simulink model "AirPmCtrl"  (R2022b)
settings: Solver=FixedStepDiscrete  StartTime=0.0  StopTime=10.0  FixedStep=1  RelTol=1e-3
settings Model: Creator=blue.ge  LastModifiedBy=blue.ge
settings DataIOCC: SaveFormat=StructureWithTime
settings OptimizationCC: DefaultParameterBehavior=Inlined  EnhancedBackFolding=on  PassReuseOutputArgsAs=Individual arguments  ZeroExternalMemoryAtStartup=off  ZeroInternalMemoryAtStartup=off  OptimizeBlockOrder=speed  DifferentSizesBufferReuse=on  OptimizationCustomize=off  EfficientTunableParamExpr=on
settings DebuggingCC: ParameterTunabilityLossMsg=error
settings ModelReferenceCC: ModelReferenceNumInstancesAllowed=Single
settings SFSimCC: MATLABDynamicMemAlloc=off
settings RTWCC: Description=AUTOSAR  SystemTargetFile=autosar.tlc  GenCodeOnly=on  TemplateMakefile=ert_default_tmf
settings CodeAppCC: ForceParamTrailComments=on  IgnoreCustomStorageClasses=off  OperatorAnnotations=on  SimulinkDataObjDesc=on  SFDataObjDesc=on  InsertBlockDesc=on
settings STFCustomTargetCC: TargetLangStandard=C99 (ISO)  MultiwordLength=256  ParMdlRefBuildCompliant=on  CompOptLevelCompliant=on  IncludeMdlTerminateFcn=off  CombineOutputUpdateFcns=on  SuppressErrorStatus=on  ERTCustomFileBanners=on  SupportNonFinite=off  SupportComplex=off  SupportContinuousTime=off  SupportNonInlinedSFcns=off  RemoveResetFunc=on  MATLABClassNameForMDSCustomization=Simulink.SoftwareTarget.ERTCustomization  AutosarCompliant=on  UseToolchainInfoCompliant=on  LookupTableObjectStructAxisOrder=2,1,3,4,...  LUTObjectStructOrderEvenSpacing=Size,Table,Breakpoints  SystemTargetFile=autosar.tlc
settings ERTTargetCC: TargetLangStandard=C99 (ISO)  ConcurrentExecutionCompliant=off  IncludeMdlTerminateFcn=off  SuppressErrorStatus=on  SupportNonFinite=off  SupportComplex=off  ModelStepFunctionPrototypeControlCompliant=off  CPPClassGenCompliant=off  AutosarCompliant=on  LookupTableObjectStructAxisOrder=2,1,3,4,...  LUTObjectStructOrderEvenSpacing=Size,Table,Breakpoints  InlinedParameterPlacement=NonHierarchical  ERTCustomFileTemplate=example_file_process.tlc

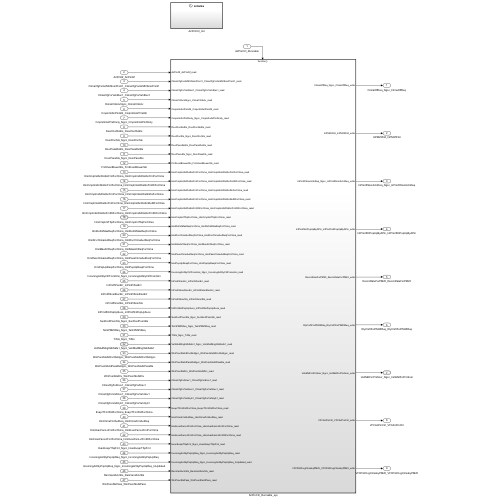
[thumbnail: Simulink's stored preview of the top-level diagram]
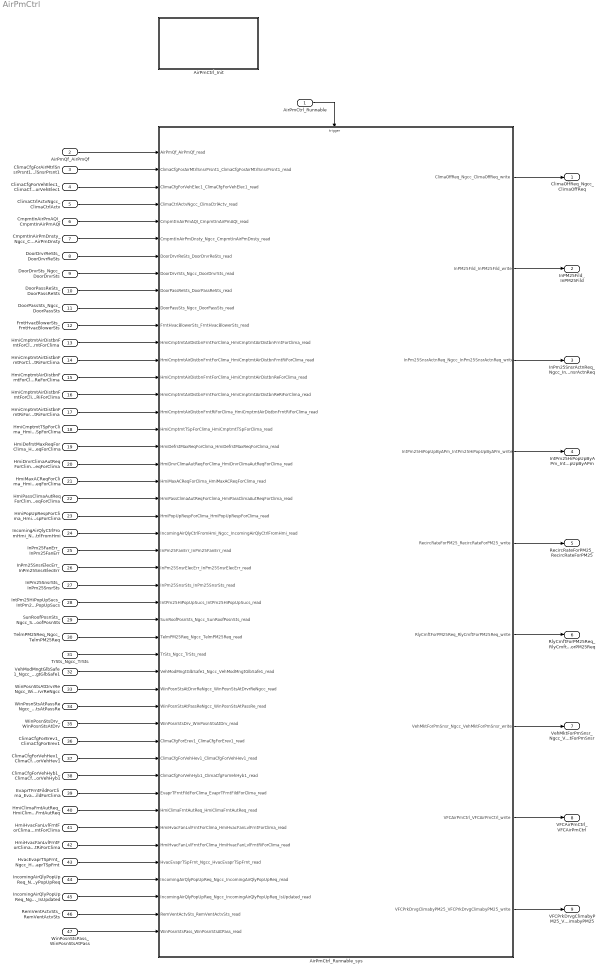
== system AirPmCtrl ==
  AirPmCtrl_Runnable : Inport  SampleTime=1  OutputFunctionCall=on
  AirPmQf_AirPmQf : Inport  Port=2  OutDataTypeStr=Enum: AirPmQf  PortDimensions=1
  ClimaCfgForAirMtrlSnsrPrsnt1_ClimaCfgForAirMtrlSnsrPrsnt1 : Inport  Port=3  OutDataTypeStr=Enum: CarCfgForAirMtrlSnsrGly  PortDimensions=1
  ClimaCfgForVehElec1_ClimaCfgForVehElec1 : Inport  Port=4  OutDataTypeStr=Boolean  PortDimensions=1
  ClimaCtrlActvNgcc_ClimaCtrlActv : Inport  Port=5  OutDataTypeStr=Boolean  PortDimensions=1
  CmpmtInAirPmAQI_CmpmtInAirPmAQI : Inport  Port=6  OutDataTypeStr=Enum: CmpmtAirPmLvl  PortDimensions=1
  CmpmtInAirPmDnsty_Ngcc_CmpmtInAirPmDnsty : Inport  Port=7  OutDataTypeStr=UInt10  PortDimensions=1
  DoorDrvrReSts_DoorDrvrReSts : Inport  Port=8  OutDataTypeStr=Enum: DoorSts2  PortDimensions=1
  DoorDrvrSts_Ngcc_DoorDrvrSts : Inport  Port=9  OutDataTypeStr=Enum: DoorSts2  PortDimensions=1
  DoorPassReSts_DoorPassReSts : Inport  Port=10  OutDataTypeStr=Enum: DoorSts2  PortDimensions=1
  DoorPassSts_Ngcc_DoorPassSts : Inport  Port=11  OutDataTypeStr=Enum: DoorSts2  PortDimensions=1
  FrntHvacBlowerSts_FrntHvacBlowerSts : Inport  Port=12  OutDataTypeStr=Enum: HvacFanSts  PortDimensions=1
  HmiCmptmtAirDistbnFrntForClima_HmiCmptmtAirDistbnFrntForClima : Inport  Port=13  OutDataTypeStr=Enum: HmiCmptmtAirDistbnFrnt  PortDimensions=1
  HmiCmptmtAirDistbnFrntForClima_HmiCmptmtAirDistbnFrntRiForClima : Inport  Port=14  OutDataTypeStr=Enum: HmiCmptmtAirDistbnFrnt  PortDimensions=1
  HmiCmptmtAirDistbnFrntForClima_HmiCmptmtAirDistbnReForClima : Inport  Port=15  OutDataTypeStr=Enum: HmiCmptmtAirDistbnFrnt  PortDimensions=1
  HmiCmptmtAirDistbnFrntForClima_HmiCmptmtAirDistbnReRiForClima : Inport  Port=16  OutDataTypeStr=Enum: HmiCmptmtAirDistbnFrnt  PortDimensions=1
  HmiCmptmtAirDistbnFrntRiForClima_HmiCmptmtAirDistbnFrntRiForClima : Inport  Port=17  OutDataTypeStr=Enum: HmiCmptmtAirDistbnFrnt  PortDimensions=1
  HmiCmptmtTSpForClima_HmiCmptmtTSpForClima : Inport  Port=18  OutDataTypeStr=Bus: HmiCmptmtTSp  PortDimensions=1  BusOutputAsStruct=on
  HmiDefrstMaxReqForClima_HmiDefrstMaxReqForClima : Inport  Port=19  OutDataTypeStr=Enum: ActrReq  PortDimensions=1
  HmiDrvrClimaAutReqForClima_HmiDrvrClimaAutReqForClima : Inport  Port=20  OutDataTypeStr=Enum: OnOff1  PortDimensions=1
  HmiMaxACReqForClima_HmiMaxACReqForClima : Inport  Port=21  OutDataTypeStr=Enum: OnOff1  PortDimensions=1
  HmiPassClimaAutReqForClima_HmiPassClimaAutReqForClima : Inport  Port=22  OutDataTypeStr=Enum: OnOff1  PortDimensions=1
  HmiPopUpRespForClima_HmiPopUpRespForClima : Inport  Port=23  OutDataTypeStr=Enum: BookChargeSetResponse  PortDimensions=1
  IncomingAirQlyCtrlFromHmi_Ngcc_IncomingAirQlyCtrlFromHmi : Inport  Port=24  OutDataTypeStr=Enum: OnOff1  PortDimensions=1
  InPm25FanErr_InPm25FanErr : Inport  Port=25  OutDataTypeStr=Enum: FanErr  PortDimensions=1
  InPm25SnsrElecErr_InPm25SnsrElecErr : Inport  Port=26  OutDataTypeStr=Enum: PmSnsrErrSts  PortDimensions=1
  InPm25SnsrSts_InPm25SnsrSts : Inport  Port=27  OutDataTypeStr=Enum: PmSnsrSts  PortDimensions=1
  IntPm25HiPopUpSucs_IntPm25HiPopUpSucs : Inport  Port=28  OutDataTypeStr=Boolean  PortDimensions=1
  SunRoofPosnSts_Ngcc_SunRoofPosnSts : Inport  Port=29  OutDataTypeStr=Enum: WinAndRoofAndCurtPosnTyp  PortDimensions=1
  TelmPM25Req_Ngcc_TelmPM25Req : Inport  Port=30  OutDataTypeStr=Enum: ReqSts1  PortDimensions=1
  TrSts_Ngcc_TrSts : Inport  Port=31  OutDataTypeStr=Enum: DoorSts2  PortDimensions=1
  VehModMngtGlbSafe1_Ngcc_VehModMngtGlbSafe1 : Inport  Port=32  OutDataTypeStr=Bus: VehModMngtGlbSafe1  PortDimensions=1  BusOutputAsStruct=on
  WinPosnStsAtDrvrReNgcc_WinPosnStsAtDrvrReNgcc : Inport  Port=33  OutDataTypeStr=Enum: WinAndRoofAndCurtPosnTyp  PortDimensions=1
  WinPosnStsAtPassReNgcc_WinPosnStsAtPassRe : Inport  Port=34  OutDataTypeStr=Enum: WinAndRoofAndCurtPosnTyp  PortDimensions=1
  WinPosnStsDrv_WinPosnStsAtDrv : Inport  Port=35  OutDataTypeStr=Enum: WinAndRoofAndCurtPosnTyp  PortDimensions=1
  ClimaCfgForErev1_ClimaCfgForErev1 : Inport  Port=36  OutDataTypeStr=Boolean  PortDimensions=1
  ClimaCfgForVehHev1_ClimaCfgForVehHev1 : Inport  Port=37  OutDataTypeStr=Boolean  PortDimensions=1
  ClimaCfgForVehHyb1_ClimaCfgForVehHyb1 : Inport  Port=38  OutDataTypeStr=Boolean  PortDimensions=1
  EvaprTFrntFildForClima_EvaprTFrntFildForClima : Inport  Port=39  OutDataTypeStr=T30  PortDimensions=1
  HmiClimaFrntAutReq_HmiClimaFrntAutReq : Inport  Port=40  OutDataTypeStr=Enum: OnOff1  PortDimensions=1
  HmiHvacFanLvlFrntForClima_HmiHvacFanLvlFrntForClima : Inport  Port=41  OutDataTypeStr=Enum: HmiHvacFanLvl  PortDimensions=1
  HmiHvacFanLvlFrntForClima_HmiHvacFanLvlFrntRiForClima : Inport  Port=42  OutDataTypeStr=Enum: HmiHvacFanLvl  PortDimensions=1
  HvacEvaprTSpFrnt_Ngcc_HvacEvaprTSpFrnt : Inport  Port=43  OutDataTypeStr=HvacEvaprTSp  PortDimensions=1
  IncomingAirQlyPopUpReq_Ngcc_IncomingAirQlyPopUpReq : Inport  Port=44  OutDataTypeStr=Enum: OnOff1  PortDimensions=1
  IncomingAirQlyPopUpReq_Ngcc_IncomingAirQlyPopUpReq_IsUpdated : Inport  Port=45  OutDataTypeStr=boolean  PortDimensions=1
  RemVentActvSts_RemVentActvSts : Inport  Port=46  OutDataTypeStr=Enum: OnOff1  PortDimensions=1
  WinPosnStsPass_WinPosnStsAtPass : Inport  Port=47  OutDataTypeStr=Enum: WinAndRoofAndCurtPosnTyp  PortDimensions=1
  AirPmCtrl_Init : Subsystem
  AirPmCtrl_Runnable_sys : Subsystem
  ClimaOffReq_Ngcc_ClimaOffReq : Outport  OutDataTypeStr=Enum: ClimaOffReq  PortDimensions=1
  InPM25Fild_InPM25Fild : Outport  Port=2  OutDataTypeStr=UInt10  PortDimensions=1
  InPm25SnsrActnReq_Ngcc_InPm25SnsrActnReq : Outport  Port=3  OutDataTypeStr=Enum: OnOff1  PortDimensions=1
  IntPm25HiPopUpByAPm_IntPm25HiPopUpByAPm : Outport  Port=4  OutDataTypeStr=Enum: IntPmHiPopUp  PortDimensions=1
  RecircRateForPM25_RecircRateForPM25 : Outport  Port=5  OutDataTypeStr=Perc30  PortDimensions=1
  RlyCmftForPM25Req_RlyCmftForPM25Req : Outport  Port=6  OutDataTypeStr=Enum: OnOff1  PortDimensions=1
  VehMktForPmSnsr_Ngcc_VehMktForPmSnsr : Outport  Port=7  OutDataTypeStr=Bus: AirQlyMeasInValReq  PortDimensions=1  BusOutputAsStruct=on
  VFCAirPmCtrl_VFCAirPmCtrl : Outport  Port=8  OutDataTypeStr=Enum: OnOff1  PortDimensions=1
  VFCPrkDrvgClimabyPM25_VFCPrkDrvgClimabyPM25 : Outport  Port=9  OutDataTypeStr=Boolean  PortDimensions=1
  wires:
  AirPmQf_AirPmQf/1 -> AirPmCtrl_Runnable_sys/1(AirPmQf_AirPmQf_read)
  ClimaCfgForAirMtrlSnsrPrsnt1_ClimaCfgForAirMtrlSnsrPrsnt1/1 -> AirPmCtrl_Runnable_sys/2(ClimaCfgForAirMtrlSnsrPrsnt1_ClimaCfgForAirMtrlSnsrPrsnt1_read)
  ClimaCfgForVehElec1_ClimaCfgForVehElec1/1 -> AirPmCtrl_Runnable_sys/3(ClimaCfgForVehElec1_ClimaCfgForVehElec1_read)
  ClimaCtrlActvNgcc_ClimaCtrlActv/1 -> AirPmCtrl_Runnable_sys/4(ClimaCtrlActvNgcc_ClimaCtrlActv_read)
  CmpmtInAirPmAQI_CmpmtInAirPmAQI/1 -> AirPmCtrl_Runnable_sys/5(CmpmtInAirPmAQI_CmpmtInAirPmAQI_read)
  CmpmtInAirPmDnsty_Ngcc_CmpmtInAirPmDnsty/1 -> AirPmCtrl_Runnable_sys/6(CmpmtInAirPmDnsty_Ngcc_CmpmtInAirPmDnsty_read)
  DoorDrvrReSts_DoorDrvrReSts/1 -> AirPmCtrl_Runnable_sys/7(DoorDrvrReSts_DoorDrvrReSts_read)
  DoorDrvrSts_Ngcc_DoorDrvrSts/1 -> AirPmCtrl_Runnable_sys/8(DoorDrvrSts_Ngcc_DoorDrvrSts_read)
  DoorPassReSts_DoorPassReSts/1 -> AirPmCtrl_Runnable_sys/9(DoorPassReSts_DoorPassReSts_read)
  DoorPassSts_Ngcc_DoorPassSts/1 -> AirPmCtrl_Runnable_sys/10(DoorPassSts_Ngcc_DoorPassSts_read)
  FrntHvacBlowerSts_FrntHvacBlowerSts/1 -> AirPmCtrl_Runnable_sys/11(FrntHvacBlowerSts_FrntHvacBlowerSts_read)
  HmiCmptmtAirDistbnFrntForClima_HmiCmptmtAirDistbnFrntForClima/1 -> AirPmCtrl_Runnable_sys/12(HmiCmptmtAirDistbnFrntForClima_HmiCmptmtAirDistbnFrntForClima_read)
  HmiCmptmtAirDistbnFrntForClima_HmiCmptmtAirDistbnFrntRiForClima/1 -> AirPmCtrl_Runnable_sys/13(HmiCmptmtAirDistbnFrntForClima_HmiCmptmtAirDistbnFrntRiForClima_read)
  HmiCmptmtAirDistbnFrntForClima_HmiCmptmtAirDistbnReForClima/1 -> AirPmCtrl_Runnable_sys/14(HmiCmptmtAirDistbnFrntForClima_HmiCmptmtAirDistbnReForClima_read)
  HmiCmptmtAirDistbnFrntForClima_HmiCmptmtAirDistbnReRiForClima/1 -> AirPmCtrl_Runnable_sys/15(HmiCmptmtAirDistbnFrntForClima_HmiCmptmtAirDistbnReRiForClima_read)
  HmiCmptmtAirDistbnFrntRiForClima_HmiCmptmtAirDistbnFrntRiForClima/1 -> AirPmCtrl_Runnable_sys/16(HmiCmptmtAirDistbnFrntRiForClima_HmiCmptmtAirDistbnFrntRiForClima_read)
  HmiCmptmtTSpForClima_HmiCmptmtTSpForClima/1 -> AirPmCtrl_Runnable_sys/17(HmiCmptmtTSpForClima_HmiCmptmtTSpForClima_read)
  HmiDefrstMaxReqForClima_HmiDefrstMaxReqForClima/1 -> AirPmCtrl_Runnable_sys/18(HmiDefrstMaxReqForClima_HmiDefrstMaxReqForClima_read)
  HmiDrvrClimaAutReqForClima_HmiDrvrClimaAutReqForClima/1 -> AirPmCtrl_Runnable_sys/19(HmiDrvrClimaAutReqForClima_HmiDrvrClimaAutReqForClima_read)
  HmiMaxACReqForClima_HmiMaxACReqForClima/1 -> AirPmCtrl_Runnable_sys/20(HmiMaxACReqForClima_HmiMaxACReqForClima_read)
  HmiPassClimaAutReqForClima_HmiPassClimaAutReqForClima/1 -> AirPmCtrl_Runnable_sys/21(HmiPassClimaAutReqForClima_HmiPassClimaAutReqForClima_read)
  HmiPopUpRespForClima_HmiPopUpRespForClima/1 -> AirPmCtrl_Runnable_sys/22(HmiPopUpRespForClima_HmiPopUpRespForClima_read)
  IncomingAirQlyCtrlFromHmi_Ngcc_IncomingAirQlyCtrlFromHmi/1 -> AirPmCtrl_Runnable_sys/23(IncomingAirQlyCtrlFromHmi_Ngcc_IncomingAirQlyCtrlFromHmi_read)
  InPm25FanErr_InPm25FanErr/1 -> AirPmCtrl_Runnable_sys/24(InPm25FanErr_InPm25FanErr_read)
  InPm25SnsrElecErr_InPm25SnsrElecErr/1 -> AirPmCtrl_Runnable_sys/25(InPm25SnsrElecErr_InPm25SnsrElecErr_read)
  InPm25SnsrSts_InPm25SnsrSts/1 -> AirPmCtrl_Runnable_sys/26(InPm25SnsrSts_InPm25SnsrSts_read)
  IntPm25HiPopUpSucs_IntPm25HiPopUpSucs/1 -> AirPmCtrl_Runnable_sys/27(IntPm25HiPopUpSucs_IntPm25HiPopUpSucs_read)
  SunRoofPosnSts_Ngcc_SunRoofPosnSts/1 -> AirPmCtrl_Runnable_sys/28(SunRoofPosnSts_Ngcc_SunRoofPosnSts_read)
  TelmPM25Req_Ngcc_TelmPM25Req/1 -> AirPmCtrl_Runnable_sys/29(TelmPM25Req_Ngcc_TelmPM25Req_read)
  TrSts_Ngcc_TrSts/1 -> AirPmCtrl_Runnable_sys/30(TrSts_Ngcc_TrSts_read)
  VehModMngtGlbSafe1_Ngcc_VehModMngtGlbSafe1/1 -> AirPmCtrl_Runnable_sys/31(VehModMngtGlbSafe1_Ngcc_VehModMngtGlbSafe1_read)
  WinPosnStsAtDrvrReNgcc_WinPosnStsAtDrvrReNgcc/1 -> AirPmCtrl_Runnable_sys/32(WinPosnStsAtDrvrReNgcc_WinPosnStsAtDrvrReNgcc_read)
  WinPosnStsAtPassReNgcc_WinPosnStsAtPassRe/1 -> AirPmCtrl_Runnable_sys/33(WinPosnStsAtPassReNgcc_WinPosnStsAtPassRe_read)
  WinPosnStsDrv_WinPosnStsAtDrv/1 -> AirPmCtrl_Runnable_sys/34(WinPosnStsDrv_WinPosnStsAtDrv_read)
  ClimaCfgForErev1_ClimaCfgForErev1/1 -> AirPmCtrl_Runnable_sys/35(ClimaCfgForErev1_ClimaCfgForErev1_read)
  ClimaCfgForVehHev1_ClimaCfgForVehHev1/1 -> AirPmCtrl_Runnable_sys/36(ClimaCfgForVehHev1_ClimaCfgForVehHev1_read)
  ClimaCfgForVehHyb1_ClimaCfgForVehHyb1/1 -> AirPmCtrl_Runnable_sys/37(ClimaCfgForVehHyb1_ClimaCfgForVehHyb1_read)
  EvaprTFrntFildForClima_EvaprTFrntFildForClima/1 -> AirPmCtrl_Runnable_sys/38(EvaprTFrntFildForClima_EvaprTFrntFildForClima_read)
  HmiClimaFrntAutReq_HmiClimaFrntAutReq/1 -> AirPmCtrl_Runnable_sys/39(HmiClimaFrntAutReq_HmiClimaFrntAutReq_read)
  HmiHvacFanLvlFrntForClima_HmiHvacFanLvlFrntForClima/1 -> AirPmCtrl_Runnable_sys/40(HmiHvacFanLvlFrntForClima_HmiHvacFanLvlFrntForClima_read)
  HmiHvacFanLvlFrntForClima_HmiHvacFanLvlFrntRiForClima/1 -> AirPmCtrl_Runnable_sys/41(HmiHvacFanLvlFrntForClima_HmiHvacFanLvlFrntRiForClima_read)
  HvacEvaprTSpFrnt_Ngcc_HvacEvaprTSpFrnt/1 -> AirPmCtrl_Runnable_sys/42(HvacEvaprTSpFrnt_Ngcc_HvacEvaprTSpFrnt_read)
  IncomingAirQlyPopUpReq_Ngcc_IncomingAirQlyPopUpReq/1 -> AirPmCtrl_Runnable_sys/43(IncomingAirQlyPopUpReq_Ngcc_IncomingAirQlyPopUpReq_read)
  IncomingAirQlyPopUpReq_Ngcc_IncomingAirQlyPopUpReq_IsUpdated/1 -> AirPmCtrl_Runnable_sys/44(IncomingAirQlyPopUpReq_Ngcc_IncomingAirQlyPopUpReq_IsUpdated_read)
  RemVentActvSts_RemVentActvSts/1 -> AirPmCtrl_Runnable_sys/45(RemVentActvSts_RemVentActvSts_read)
  WinPosnStsPass_WinPosnStsAtPass/1 -> AirPmCtrl_Runnable_sys/46(WinPosnStsPass_WinPosnStsAtPass_read)
  AirPmCtrl_Runnable_sys/1(ClimaOffReq_Ngcc_ClimaOffReq_write) -> ClimaOffReq_Ngcc_ClimaOffReq/1
  AirPmCtrl_Runnable_sys/2(InPM25Fild_InPM25Fild_write) -> InPM25Fild_InPM25Fild/1
  AirPmCtrl_Runnable_sys/3(InPm25SnsrActnReq_Ngcc_InPm25SnsrActnReq_write) -> InPm25SnsrActnReq_Ngcc_InPm25SnsrActnReq/1
  AirPmCtrl_Runnable_sys/4(IntPm25HiPopUpByAPm_IntPm25HiPopUpByAPm_write) -> IntPm25HiPopUpByAPm_IntPm25HiPopUpByAPm/1
  AirPmCtrl_Runnable_sys/5(RecircRateForPM25_RecircRateForPM25_write) -> RecircRateForPM25_RecircRateForPM25/1
  AirPmCtrl_Runnable_sys/6(RlyCmftForPM25Req_RlyCmftForPM25Req_write) -> RlyCmftForPM25Req_RlyCmftForPM25Req/1
  AirPmCtrl_Runnable_sys/7(VehMktForPmSnsr_Ngcc_VehMktForPmSnsr_write) -> VehMktForPmSnsr_Ngcc_VehMktForPmSnsr/1
  AirPmCtrl_Runnable_sys/8(VFCAirPmCtrl_VFCAirPmCtrl_write) -> VFCAirPmCtrl_VFCAirPmCtrl/1
  AirPmCtrl_Runnable_sys/9(VFCPrkDrvgClimabyPM25_VFCPrkDrvgClimabyPM25_write) -> VFCPrkDrvgClimabyPM25_VFCPrkDrvgClimabyPM25/1
  AirPmCtrl_Runnable/1 -> AirPmCtrl_Runnable_sys/Trigger

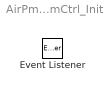
== system AirPmCtrl/AirPmCtrl_Init ==
  Event Listener : EventListener  EventName=reset

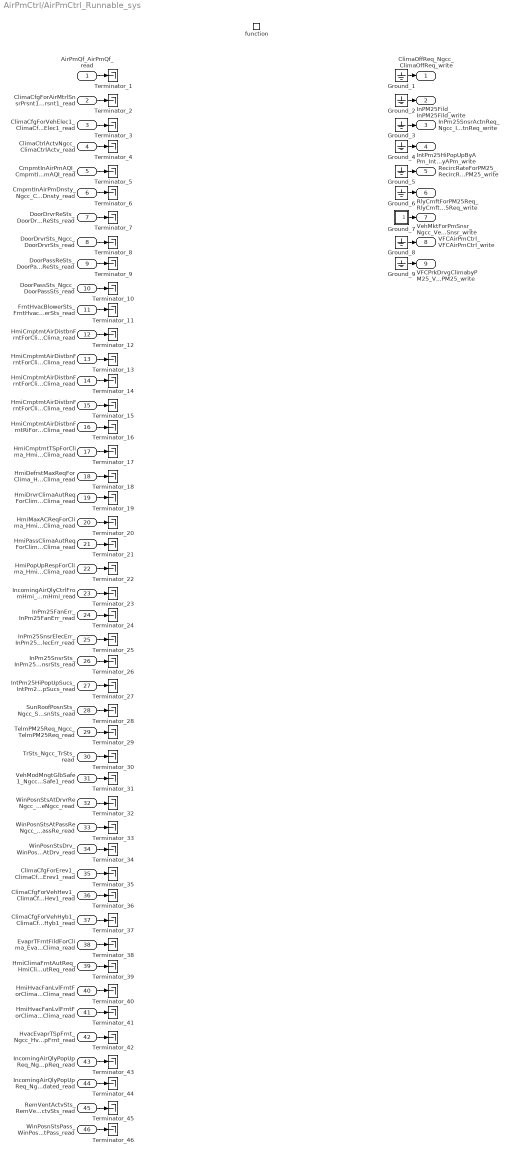
== system AirPmCtrl/AirPmCtrl_Runnable_sys ==
  AirPmQf_AirPmQf_read : Inport
  ClimaCfgForAirMtrlSnsrPrsnt1_ClimaCfgForAirMtrlSnsrPrsnt1_read : Inport  Port=2
  ClimaCfgForVehElec1_ClimaCfgForVehElec1_read : Inport  Port=3
  ClimaCtrlActvNgcc_ClimaCtrlActv_read : Inport  Port=4
  CmpmtInAirPmAQI_CmpmtInAirPmAQI_read : Inport  Port=5
  CmpmtInAirPmDnsty_Ngcc_CmpmtInAirPmDnsty_read : Inport  Port=6
  DoorDrvrReSts_DoorDrvrReSts_read : Inport  Port=7
  DoorDrvrSts_Ngcc_DoorDrvrSts_read : Inport  Port=8
  DoorPassReSts_DoorPassReSts_read : Inport  Port=9
  DoorPassSts_Ngcc_DoorPassSts_read : Inport  Port=10
  FrntHvacBlowerSts_FrntHvacBlowerSts_read : Inport  Port=11
  HmiCmptmtAirDistbnFrntForClima_HmiCmptmtAirDistbnFrntForClima_read : Inport  Port=12
  HmiCmptmtAirDistbnFrntForClima_HmiCmptmtAirDistbnFrntRiForClima_read : Inport  Port=13
  HmiCmptmtAirDistbnFrntForClima_HmiCmptmtAirDistbnReForClima_read : Inport  Port=14
  HmiCmptmtAirDistbnFrntForClima_HmiCmptmtAirDistbnReRiForClima_read : Inport  Port=15
  HmiCmptmtAirDistbnFrntRiForClima_HmiCmptmtAirDistbnFrntRiForClima_read : Inport  Port=16
  HmiCmptmtTSpForClima_HmiCmptmtTSpForClima_read : Inport  Port=17
  HmiDefrstMaxReqForClima_HmiDefrstMaxReqForClima_read : Inport  Port=18
  HmiDrvrClimaAutReqForClima_HmiDrvrClimaAutReqForClima_read : Inport  Port=19
  HmiMaxACReqForClima_HmiMaxACReqForClima_read : Inport  Port=20
  HmiPassClimaAutReqForClima_HmiPassClimaAutReqForClima_read : Inport  Port=21
  HmiPopUpRespForClima_HmiPopUpRespForClima_read : Inport  Port=22
  IncomingAirQlyCtrlFromHmi_Ngcc_IncomingAirQlyCtrlFromHmi_read : Inport  Port=23
  InPm25FanErr_InPm25FanErr_read : Inport  Port=24
  InPm25SnsrElecErr_InPm25SnsrElecErr_read : Inport  Port=25
  InPm25SnsrSts_InPm25SnsrSts_read : Inport  Port=26
  IntPm25HiPopUpSucs_IntPm25HiPopUpSucs_read : Inport  Port=27
  SunRoofPosnSts_Ngcc_SunRoofPosnSts_read : Inport  Port=28
  TelmPM25Req_Ngcc_TelmPM25Req_read : Inport  Port=29
  TrSts_Ngcc_TrSts_read : Inport  Port=30
  VehModMngtGlbSafe1_Ngcc_VehModMngtGlbSafe1_read : Inport  Port=31
  WinPosnStsAtDrvrReNgcc_WinPosnStsAtDrvrReNgcc_read : Inport  Port=32
  WinPosnStsAtPassReNgcc_WinPosnStsAtPassRe_read : Inport  Port=33
  WinPosnStsDrv_WinPosnStsAtDrv_read : Inport  Port=34
  ClimaCfgForErev1_ClimaCfgForErev1_read : Inport  Port=35
  ClimaCfgForVehHev1_ClimaCfgForVehHev1_read : Inport  Port=36
  ClimaCfgForVehHyb1_ClimaCfgForVehHyb1_read : Inport  Port=37
  EvaprTFrntFildForClima_EvaprTFrntFildForClima_read : Inport  Port=38
  HmiClimaFrntAutReq_HmiClimaFrntAutReq_read : Inport  Port=39
  HmiHvacFanLvlFrntForClima_HmiHvacFanLvlFrntForClima_read : Inport  Port=40
  HmiHvacFanLvlFrntForClima_HmiHvacFanLvlFrntRiForClima_read : Inport  Port=41
  HvacEvaprTSpFrnt_Ngcc_HvacEvaprTSpFrnt_read : Inport  Port=42
  IncomingAirQlyPopUpReq_Ngcc_IncomingAirQlyPopUpReq_read : Inport  Port=43
  IncomingAirQlyPopUpReq_Ngcc_IncomingAirQlyPopUpReq_IsUpdated_read : Inport  Port=44
  RemVentActvSts_RemVentActvSts_read : Inport  Port=45
  WinPosnStsPass_WinPosnStsAtPass_read : Inport  Port=46
  function : TriggerPort  TriggerType=function-call  VariantControl=(inherit)  SampleTimeType=periodic  SampleTime=-1
  Ground_{1..6,8..9} (8 alike) : Ground
  Ground_7 : Subsystem
  Terminator_{1..46} (46 alike) : Terminator
  ClimaOffReq_Ngcc_ClimaOffReq_write : Outport
      settings: EnsureOutportIsVirtual=on
  InPM25Fild_InPM25Fild_write : Outport  Port=2
      settings: EnsureOutportIsVirtual=on
  InPm25SnsrActnReq_Ngcc_InPm25SnsrActnReq_write : Outport  Port=3
      settings: EnsureOutportIsVirtual=on
  IntPm25HiPopUpByAPm_IntPm25HiPopUpByAPm_write : Outport  Port=4
      settings: EnsureOutportIsVirtual=on
  RecircRateForPM25_RecircRateForPM25_write : Outport  Port=5
      settings: EnsureOutportIsVirtual=on
  RlyCmftForPM25Req_RlyCmftForPM25Req_write : Outport  Port=6
      settings: EnsureOutportIsVirtual=on
  VehMktForPmSnsr_Ngcc_VehMktForPmSnsr_write : Outport  Port=7
      settings: EnsureOutportIsVirtual=on
  VFCAirPmCtrl_VFCAirPmCtrl_write : Outport  Port=8
      settings: EnsureOutportIsVirtual=on
  VFCPrkDrvgClimabyPM25_VFCPrkDrvgClimabyPM25_write : Outport  Port=9
      settings: EnsureOutportIsVirtual=on
  wires:
  AirPmQf_AirPmQf_read/1 -> Terminator_1/1
  ClimaCfgForAirMtrlSnsrPrsnt1_ClimaCfgForAirMtrlSnsrPrsnt1_read/1 -> Terminator_2/1
  ClimaCfgForVehElec1_ClimaCfgForVehElec1_read/1 -> Terminator_3/1
  ClimaCtrlActvNgcc_ClimaCtrlActv_read/1 -> Terminator_4/1
  CmpmtInAirPmAQI_CmpmtInAirPmAQI_read/1 -> Terminator_5/1
  CmpmtInAirPmDnsty_Ngcc_CmpmtInAirPmDnsty_read/1 -> Terminator_6/1
  DoorDrvrReSts_DoorDrvrReSts_read/1 -> Terminator_7/1
  DoorDrvrSts_Ngcc_DoorDrvrSts_read/1 -> Terminator_8/1
  DoorPassReSts_DoorPassReSts_read/1 -> Terminator_9/1
  DoorPassSts_Ngcc_DoorPassSts_read/1 -> Terminator_10/1
  FrntHvacBlowerSts_FrntHvacBlowerSts_read/1 -> Terminator_11/1
  HmiCmptmtAirDistbnFrntForClima_HmiCmptmtAirDistbnFrntForClima_read/1 -> Terminator_12/1
  HmiCmptmtAirDistbnFrntForClima_HmiCmptmtAirDistbnFrntRiForClima_read/1 -> Terminator_13/1
  HmiCmptmtAirDistbnFrntForClima_HmiCmptmtAirDistbnReForClima_read/1 -> Terminator_14/1
  HmiCmptmtAirDistbnFrntForClima_HmiCmptmtAirDistbnReRiForClima_read/1 -> Terminator_15/1
  HmiCmptmtAirDistbnFrntRiForClima_HmiCmptmtAirDistbnFrntRiForClima_read/1 -> Terminator_16/1
  HmiCmptmtTSpForClima_HmiCmptmtTSpForClima_read/1 -> Terminator_17/1
  HmiDefrstMaxReqForClima_HmiDefrstMaxReqForClima_read/1 -> Terminator_18/1
  HmiDrvrClimaAutReqForClima_HmiDrvrClimaAutReqForClima_read/1 -> Terminator_19/1
  HmiMaxACReqForClima_HmiMaxACReqForClima_read/1 -> Terminator_20/1
  HmiPassClimaAutReqForClima_HmiPassClimaAutReqForClima_read/1 -> Terminator_21/1
  HmiPopUpRespForClima_HmiPopUpRespForClima_read/1 -> Terminator_22/1
  IncomingAirQlyCtrlFromHmi_Ngcc_IncomingAirQlyCtrlFromHmi_read/1 -> Terminator_23/1
  InPm25FanErr_InPm25FanErr_read/1 -> Terminator_24/1
  InPm25SnsrElecErr_InPm25SnsrElecErr_read/1 -> Terminator_25/1
  InPm25SnsrSts_InPm25SnsrSts_read/1 -> Terminator_26/1
  IntPm25HiPopUpSucs_IntPm25HiPopUpSucs_read/1 -> Terminator_27/1
  SunRoofPosnSts_Ngcc_SunRoofPosnSts_read/1 -> Terminator_28/1
  TelmPM25Req_Ngcc_TelmPM25Req_read/1 -> Terminator_29/1
  TrSts_Ngcc_TrSts_read/1 -> Terminator_30/1
  VehModMngtGlbSafe1_Ngcc_VehModMngtGlbSafe1_read/1 -> Terminator_31/1
  WinPosnStsAtDrvrReNgcc_WinPosnStsAtDrvrReNgcc_read/1 -> Terminator_32/1
  WinPosnStsAtPassReNgcc_WinPosnStsAtPassRe_read/1 -> Terminator_33/1
  WinPosnStsDrv_WinPosnStsAtDrv_read/1 -> Terminator_34/1
  ClimaCfgForErev1_ClimaCfgForErev1_read/1 -> Terminator_35/1
  ClimaCfgForVehHev1_ClimaCfgForVehHev1_read/1 -> Terminator_36/1
  ClimaCfgForVehHyb1_ClimaCfgForVehHyb1_read/1 -> Terminator_37/1
  EvaprTFrntFildForClima_EvaprTFrntFildForClima_read/1 -> Terminator_38/1
  HmiClimaFrntAutReq_HmiClimaFrntAutReq_read/1 -> Terminator_39/1
  HmiHvacFanLvlFrntForClima_HmiHvacFanLvlFrntForClima_read/1 -> Terminator_40/1
  HmiHvacFanLvlFrntForClima_HmiHvacFanLvlFrntRiForClima_read/1 -> Terminator_41/1
  HvacEvaprTSpFrnt_Ngcc_HvacEvaprTSpFrnt_read/1 -> Terminator_42/1
  IncomingAirQlyPopUpReq_Ngcc_IncomingAirQlyPopUpReq_read/1 -> Terminator_43/1
  IncomingAirQlyPopUpReq_Ngcc_IncomingAirQlyPopUpReq_IsUpdated_read/1 -> Terminator_44/1
  RemVentActvSts_RemVentActvSts_read/1 -> Terminator_45/1
  WinPosnStsPass_WinPosnStsAtPass_read/1 -> Terminator_46/1
  Ground_1/1 -> ClimaOffReq_Ngcc_ClimaOffReq_write/1
  Ground_2/1 -> InPM25Fild_InPM25Fild_write/1
  Ground_3/1 -> InPm25SnsrActnReq_Ngcc_InPm25SnsrActnReq_write/1
  Ground_4/1 -> IntPm25HiPopUpByAPm_IntPm25HiPopUpByAPm_write/1
  Ground_5/1 -> RecircRateForPM25_RecircRateForPM25_write/1
  Ground_6/1 -> RlyCmftForPM25Req_RlyCmftForPM25Req_write/1
  Ground_7/1(Out1) -> VehMktForPmSnsr_Ngcc_VehMktForPmSnsr_write/1
  Ground_8/1 -> VFCAirPmCtrl_VFCAirPmCtrl_write/1
  Ground_9/1 -> VFCPrkDrvgClimabyPM25_VFCPrkDrvgClimabyPM25_write/1

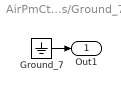
== system AirPmCtrl/AirPmCtrl_Runnable_sys/Ground_7 ==
  Ground_7 : Ground
  Out1 : Outport  OutDataTypeStr=Bus: AirQlyMeasInValReq  PortDimensions=1
  wires:
  Ground_7/1 -> Out1/1
